simulink model "non_linear_MBS_final"  (R2021b)
settings: Solver=ode15s  StartTime=0  StopTime=30  FixedStep=.00001  RelTol=1e-3
values from neighbouring files (symbols this model uses):
  A_ratio = 1.41    [non_linear_param_MBS_PF_final.m]    (also in non_linear_param_final.m)
  Annulus_area = Bore_area-Rod_area    (= 2356.19449019)    [non_linear_param_MBS_PF_final.m]    (also in non_linear_param_final.m)
  beta_F = 12000e5    (= 1200000000)    [non_linear_param_MBS_PF_final.m]    (also in non_linear_param_final.m)
  Bore_area = pi/4*Bore_diameter^2    (= 3318.30724035)    [non_linear_param_MBS_PF_final.m]    (also in non_linear_param_final.m)
  cyl_c_end = 9e8    (= 900000000)    [non_linear_param_MBS_PF_final.m]    (also in non_linear_param_final.m)
  cyl_c_visc = 1500    [non_linear_param_MBS_PF_final.m]    (also in non_linear_param_final.m)
  cyl_CH_stat = 1/50    (= 0.02)    [non_linear_param_MBS_PF_final.m]    (also in non_linear_param_final.m)
  cyl_F_coul = 75    [non_linear_param_MBS_PF_final.m]    (also in non_linear_param_final.m)
  cyl_FH_stat = 500    [non_linear_param_MBS_PF_final.m]    (also in non_linear_param_final.m)
  cyl_gamma = 250    [non_linear_param_MBS_PF_final.m]    (also in non_linear_param_final.m)
  cyl_k_end = 6e9    (= 6000000000)    [non_linear_param_MBS_PF_final.m]    (also in non_linear_param_final.m)
  cyl_KLi = 0    [non_linear_param_MBS_PF_final.m]    (also in non_linear_param_final.m)
  d_eff_long = sqrt(2*J_sec_long*k_eff_long)    [non_linear_param_MBS_PF_final.m]    (also in non_linear_param_final.m)
  d_eff_short = sqrt(2*J_sec_short*k_eff_short)    [non_linear_param_MBS_PF_final.m]    (also in non_linear_param_final.m)
  DB = 0    [non_linear_param_MBS_PF_final.m]    (also in non_linear_param_final.m = -0.114)
  DR = 1    [non_linear_param_MBS_PF_final.m]    (also in non_linear_param_final.m)
  dr_dcv = 0.7    [non_linear_param_MBS_PF_final.m]    (also in non_linear_param_final.m)
  Eff_Bulk_On = 1    [non_linear_param_MBS_PF_final.m]    (also in non_linear_param_final.m)
  eta_air = 0.7e-2    (= 0.007)    [non_linear_param_MBS_PF_final.m]    (also in non_linear_param_final.m)
  k_cv = 15    [non_linear_param_MBS_PF_final.m]    (also in non_linear_param_final.m)
  k_DCV_PA = 7.85    [non_linear_param_MBS_PF_final.m]    (also in non_linear_param_final.m)
  k_DCV_PB = 7.55    [non_linear_param_MBS_PF_final.m]    (also in non_linear_param_final.m)
  k_DCV_T = 7.85    [non_linear_param_MBS_PF_final.m]    (also in non_linear_param_final.m)
  k_eff_long = E*I/I_sec_long    (= 3050381.94041)    [non_linear_param_MBS_PF_final.m]    (also in non_linear_param_final.m)
  k_eff_short = E*I/I_sec_short    (= 13451432.5427)    [non_linear_param_MBS_PF_final.m]    (also in non_linear_param_final.m)
  k_ocv = 15    [non_linear_param_MBS_PF_final.m]    (also in non_linear_param_final.m)
  K_oil = 1.5e4*0+12000    (= 12000)    [non_linear_param_MBS_PF_final.m]    (also in non_linear_param_final.m)
  k_tanh = 0.005    [non_linear_param_MBS_PF_final.m]    (also in non_linear_param_final.m)
  kappa_air = 1.4    [non_linear_param_MBS_PF_final.m]    (also in non_linear_param_final.m)
  KR = 1    [non_linear_param_MBS_PF_final.m]    (also in non_linear_param_final.m)
  mu_ocv = 10    [non_linear_param_MBS_PF_final.m]    (also in non_linear_param_final.m = 3)
  OL_pa = -0.114    [non_linear_param_MBS_PF_final.m]    (also in non_linear_param_final.m)
  OL_pb = 0.114    [non_linear_param_MBS_PF_final.m]    (also in non_linear_param_final.m)
  p0_A = 62.79    [non_linear_param_MBS_PF_final.m]    (also in non_linear_param_final.m)
  p0_A1 = 1.08    [non_linear_param_MBS_PF_final.m]    (also in non_linear_param_final.m)
  p0_B = 1.213    [non_linear_param_MBS_PF_final.m]    (also in non_linear_param_final.m)
  p_cv_fo = 1    [non_linear_param_MBS_PF_final.m]    (also in non_linear_param_final.m)
  p_cv_set = 2.8    [non_linear_param_MBS_PF_final.m]    (also in non_linear_param_final.m)
  p_ocv_fo = 350    [non_linear_param_MBS_PF_final.m]    (also in non_linear_param_final.m)
  p_ocv_set = 196    [non_linear_param_MBS_PF_final.m]    (also in non_linear_param_final.m = 150)
  p_pc_set = 7    [non_linear_param_MBS_PF_final.m]    (also in non_linear_param_final.m)
  p_R = 1.1    [non_linear_param_MBS_PF_final.m]    (also in non_linear_param_final.m)
  p_S = 180    [non_linear_param_MBS_PF_final.m]    (also in non_linear_param_final.m)
  patm = 1.013e5    (= 101300)    [non_linear_param_MBS_PF_final.m]    (also in non_linear_param_final.m)
  Stroke = 500    [non_linear_param_MBS_PF_final.m]    (also in non_linear_param_final.m)
  t0 = 3    [non_linear_param_MBS_PF_final.m]    (also in non_linear_param_final.m = 1)
  tau_ocv = 0.0889*0+0.001    (= 0.001)    [non_linear_param_MBS_PF_final.m]    (also in non_linear_param_final.m)
  tau_pc = tau_ocv    (= 0.001)    [non_linear_param_MBS_PF_final.m]    (also in non_linear_param_final.m)
  tStepDown = 4.7*0+7.5    (= 7.5)    [non_linear_param_MBS_PF_final.m]    (also in non_linear_param_final.m = 4.6)
  tStepUp = 10.0    (= 10)    [non_linear_param_MBS_PF_final.m]    (also in non_linear_param_final.m = 6.2)
  tWait = 5.0    (= 5)    [non_linear_param_MBS_PF_final.m]    (also in non_linear_param_final.m = 8)
  UL_at = -0.05    [non_linear_param_MBS_PF_final.m]    (also in non_linear_param_final.m)
  UL_bt = 0.05    [non_linear_param_MBS_PF_final.m]    (also in non_linear_param_final.m)
  Up = 1    [non_linear_param_MBS_PF_final.m]    (also in non_linear_param_final.m)
  uS_sp = -0.4    [non_linear_param_MBS_PF_final.m]    (also in non_linear_param_final.m = -0.55)
  V_CH_A = 1.0e-03    (= 0.001)    [non_linear_param_MBS_PF_final.m]    (also in non_linear_param_final.m)
  V_CH_A1 = 0.1e-03    (= 0.0001)    [non_linear_param_MBS_PF_final.m]    (also in non_linear_param_final.m)
  V_CH_B = 1.25e-03    (= 0.00125)    [non_linear_param_MBS_PF_final.m]    (also in non_linear_param_final.m)
  wn_dcv = 30    [non_linear_param_MBS_PF_final.m]    (also in non_linear_param_final.m)
undefined symbols (not defined in the file or in its folder): sigbuilder_block, smiData, uS0, X, Y, Z
libraries: sm_lib x189, nesl_utility x4, eeGeneralControl x2
(part 1 of 6)
settings Model: Creator=Zygis  LastModifiedBy=danielh
settings SolverCC: ZeroCrossAlgorithm=Adaptive  AlgebraicLoopSolver=TrustRegion
settings DataIOCC: ExternalInput=getinputstruct(report1)  InitialState=getstatestruct(report1)  ReturnWorkspaceOutputs=off  Refine=2
settings DebuggingCC: IgnoredZcDiagnostic=none  UniqueDataStoreMsg=error  SFMachineParentedDataDiag=warning  UnderSpecifiedDimensionMsg=warning  ArithmeticOperatorsInVariantConditions=warning
settings HardwareCC: TargetBitPerPointer=64  TargetBitPerSizeT=64  TargetBitPerPtrDiffT=64  TargetLongLongMode=on  TargetIntDivRoundTo=Zero  TargetEndianess=LittleEndian  TargetWordSize=64  TargetHWDeviceType=MATLAB Host  ProdEqTarget=off
settings SFSimCC: SimUseLocalCustomCode=off  CustomCodeUndefinedFunction=UseInterfaceOnly
settings RTWCC: Description=Rapid Simulation Target  SystemTargetFile=rsim.tlc  TemplateMakefile=rsim_default_tmf  GenerateReport=on  RTWUseLocalCustomCode=off
settings RSimTargetCC: TargetLangStandard=C99 (ISO)
settings DiagnosticsConfigSet: SimMechanicsJointTargetOverSpecification=warning

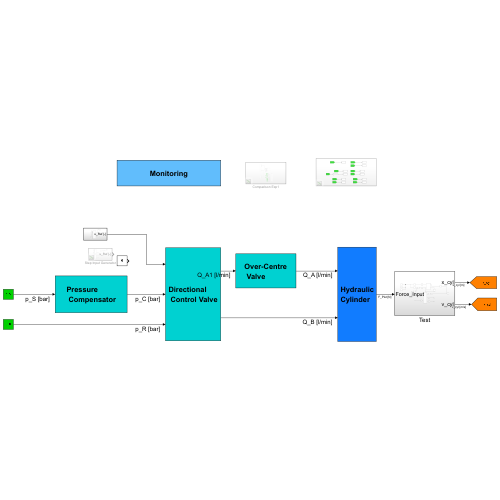
[thumbnail: Simulink's stored preview of the top-level diagram]
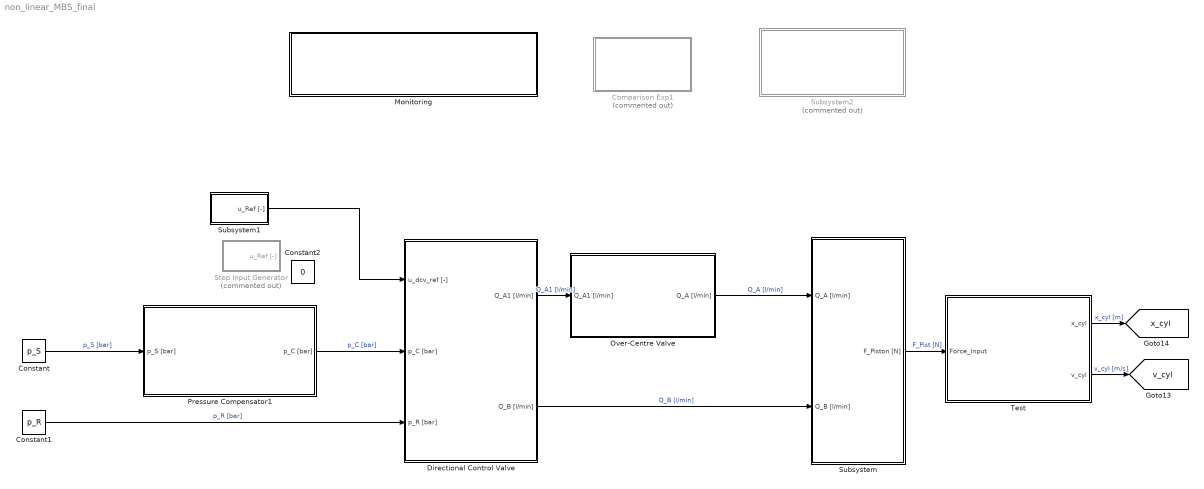
== system non_linear_MBS_final ==
  Comparison Exp1 : Subsystem  Commented=on
  Constant : Constant  Value=p_S
  Constant1 : Constant  Value=p_R
  Constant2 : Constant  Value=0
  Directional Control Valve : Subsystem
      mask Display: disp('{\bf\fontsize{22}Directional\newline Control Valve}', 'texmode','on');
  Monitoring : Subsystem
      mask Display: disp('{\bf\fontsize{22}Monitoring}', 'texmode','on');
  Over-Centre Valve : Subsystem
      mask Display: disp('{\bf\fontsize{22}Over-Centre\newline Valve}', 'texmode','on');
  Pressure Compensator1 : Subsystem
      mask Display: disp('{\bf\fontsize{22}Pressure\newline Compensator}', 'texmode','on');
  Step Input Generator : Subsystem  Commented=on
  Subsystem : Subsystem
      mask Display: disp('{\bf\fontsize{22} Hydraulic \newline Cylinder}', 'texmode','on');
  Subsystem1 : Subsystem
  Subsystem2 : Subsystem  Commented=on
  Test : Subsystem  VariantControl=Flexible_model
  tag scope: v_cyl=global, x_cyl=global
  wires:
  Over-Centre Valve/1(Q_A [l/min]) -> Subsystem/1(Q_A [l/min])  "Q_A [l/min]"
  Directional Control Valve/1(Q_A1 [l/min]) -> Over-Centre Valve/1(Q_A1 [l/min])  "Q_A1 [l/min]"
  Constant/1 -> Pressure Compensator1/1(p_S [bar])  "p_S [bar]"
  Directional Control Valve/2(Q_B [l/min]) -> Subsystem/2(Q_B [l/min])  "Q_B [l/min]"
  Constant1/1 -> Directional Control Valve/3(p_R [bar])  "p_R [bar]"
  Pressure Compensator1/1(p_C [bar]) -> Directional Control Valve/2(p_C [bar])  "p_C [bar]"
  Test/1(x_cyl) -> [x_cyl]  "x_cyl [m]"
  Test/2(v_cyl) -> [v_cyl]  "v_cyl [m/s]"
  Subsystem/1(F_Piston [N]) -> Test/1(Force_Input)  "F_Pist [N]"
  Subsystem1/1(u_Ref [-]) -> Directional Control Valve/1(u_dcv_ref [-])

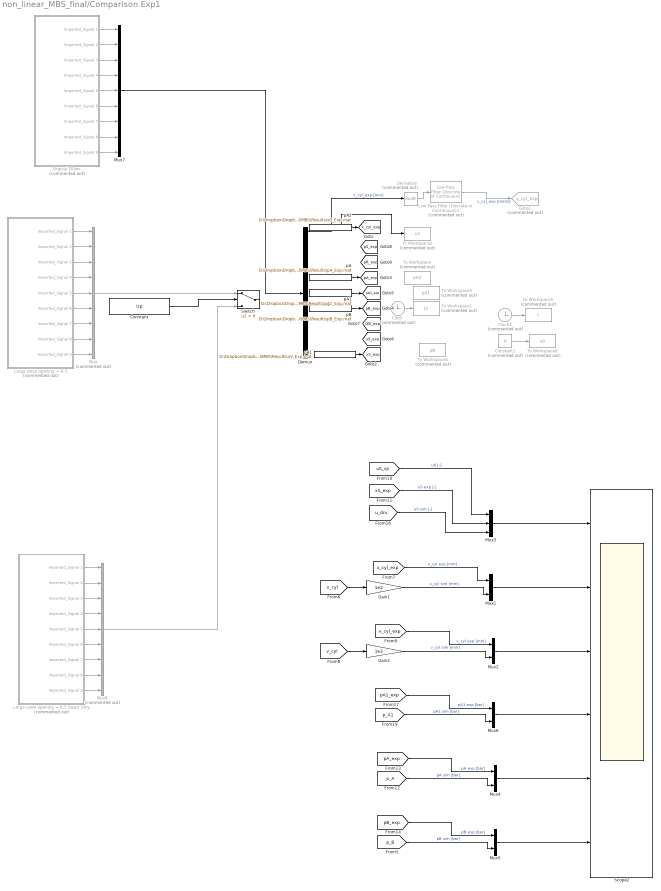
== system non_linear_MBS_final/Comparison Exp1 ==
  Clock : Clock  Commented=on
  Clock1 : Clock  Commented=on
  Constant : Constant  Value=Up
  Constant1 : Constant  Commented=on  Value=0
  Demux : Demux  Outputs=9
  Derivative : Derivative  Commented=on
  Gain1 : Gain  Gain=1e3
  Gain2 : Gain  Gain=1e3
  Goto1 : Goto  Commented=on  GotoTag=v_cyl_exp  TagVisibility=global
  Large valve opening = 0.5 : Subsystem  Tag=STV Subsys  Commented=on  LoadFcn=sigbuilder_block('load');  StartFcn=sigbuilder_block('start');  StopFcn=sigbuilder_block('stop');  ClipboardFcn=sigbuilder_block('clipboard');  mask(Sigbuilder block){tuvar=[0:10]}
      mask help: Simulink:masks:SigbuilderBlock_MD
      mask init:
      | if ~strcmp(get_param(bdroot(gcbh),'SimulationStatus'),'stopped') tuvar = sigbuilder_block('maskInit'); end
      settings: CopyFcn=sigbuilder_block('copy');  DeleteFcn=sigbuilder_block('delete');  ModelCloseFcn=sigbuilder_block('modelClose');  PreSaveFcn=sigbuilder_block('preSave');  PauseFcn=sigbuilder_block('pause');  ContinueFcn=sigbuilder_block('continue');  NameChangeFcn=sigbuilder_block('namechange');  OpenFcn=sigbuilder_block('open',[41.25 0 1392.75 801.75 ]);  CloseFcn=sigbuilder_block('close');
      mask Display: plot(0, 0, 100, 100,[2, 2, 32, 32, 2], [68, 8, 8, 68, 68],[32, 2], [38, 38], [32, 19, 2],[53, 60, 44], [32, 17, 17, 2],[16, 16, 31, 31]);txt = getActiveGroup(gcbh);text(2, 100, txt,'verticalAlignment', 'top');
  Large valve opening = 0.5 Down Only : Subsystem  Tag=STV Subsys  Commented=on  LoadFcn=sigbuilder_block('load');  StartFcn=sigbuilder_block('start');  StopFcn=sigbuilder_block('stop');  ClipboardFcn=sigbuilder_block('clipboard');  mask(Sigbuilder block){tuvar=[0:10]}
      mask help: Simulink:masks:SigbuilderBlock_MD
      mask init:
      | if ~strcmp(get_param(bdroot(gcbh),'SimulationStatus'),'stopped') tuvar = sigbuilder_block('maskInit'); end
      settings: CopyFcn=sigbuilder_block('copy');  DeleteFcn=sigbuilder_block('delete');  ModelCloseFcn=sigbuilder_block('modelClose');  PreSaveFcn=sigbuilder_block('preSave');  PauseFcn=sigbuilder_block('pause');  ContinueFcn=sigbuilder_block('continue');  NameChangeFcn=sigbuilder_block('namechange');  OpenFcn=sigbuilder_block('open',[41.25 0 1392.75 801.75 ]);  CloseFcn=sigbuilder_block('close');
      mask Display: plot(0, 0, 100, 100,[2, 2, 32, 32, 2], [68, 8, 8, 68, 68],[32, 2], [38, 38], [32, 19, 2],[53, 60, 44], [32, 17, 17, 2],[16, 16, 31, 31]);txt = getActiveGroup(gcbh);text(2, 100, txt,'verticalAlignment', 'top');
  Low-Pass Filter (Discrete or Continuous)2 : eeGeneralControl/Low-Pass Filter (Discrete or Continuous)  Commented=on  K=1  T=0.05  B=150  A=-150  init_option=Inherited from block input  x0=0  Ts=0.001
  Mux : Mux  Commented=on  Inputs=9
  Mux{1..2,4..6} (5 alike) : Mux  Inputs=2
  Mux3 : Mux  Inputs=3
  Mux7 : Mux  Inputs=9
  Mux8 : Mux  Commented=on  Inputs=9
  Orginal DOwn : Subsystem  Tag=STV Subsys  Commented=on  LoadFcn=sigbuilder_block('load');  StartFcn=sigbuilder_block('start');  StopFcn=sigbuilder_block('stop');  ClipboardFcn=sigbuilder_block('clipboard');  mask(Sigbuilder block){tuvar=[0:10]}
      mask help: Simulink:masks:SigbuilderBlock_MD
      mask init:
      | if ~strcmp(get_param(bdroot(gcbh),'SimulationStatus'),'stopped') tuvar = sigbuilder_block('maskInit'); end
      settings: CopyFcn=sigbuilder_block('copy');  DeleteFcn=sigbuilder_block('delete');  ModelCloseFcn=sigbuilder_block('modelClose');  PreSaveFcn=sigbuilder_block('preSave');  PauseFcn=sigbuilder_block('pause');  ContinueFcn=sigbuilder_block('continue');  NameChangeFcn=sigbuilder_block('namechange');  OpenFcn=sigbuilder_block('open',[437.6 199.2 550.4 400 ]);  CloseFcn=sigbuilder_block('close');
      mask Display: plot(0, 0, 100, 100,[2, 2, 32, 32, 2], [68, 8, 8, 68, 68],[32, 2], [38, 38], [32, 19, 2],[53, 60, 44], [32, 17, 17, 2],[16, 16, 31, 31]);txt = getActiveGroup(gcbh);text(2, 100, txt,'verticalAlignment', 'top');
  Scope2 : Scope  NumInputPorts=6
  Switch : Switch  Criteria=u2 > Threshold
  To Workspace : ToWorkspace  Commented=on  VariableName=pA2  SampleTime=0.001
      settings: Save2DSignal=2-D array (concatenate along first dimension)
  To Workspace1 : ToWorkspace  Commented=on  VariableName=t2  SampleTime=0.001
      settings: Save2DSignal=2-D array (concatenate along first dimension)
  To Workspace2 : ToWorkspace  Commented=on  VariableName=x2  SampleTime=0.001
      settings: Save2DSignal=2-D array (concatenate along first dimension)
  To Workspace3 : ToWorkspace  Commented=on  VariableName=pA1  SampleTime=0.001
      settings: Save2DSignal=2-D array (concatenate along first dimension)
  To Workspace4 : ToWorkspace  Commented=on  VariableName=pB  SampleTime=0.001
      settings: Save2DSignal=2-D array (concatenate along first dimension)
  To Workspace5 : ToWorkspace  Commented=on  VariableName=t  SampleTime=0.001
      settings: Save2DSignal=2-D array (concatenate along first dimension)
  To Workspace6 : ToWorkspace  Commented=on  VariableName=v0  SampleTime=0.001
      settings: Save2DSignal=2-D array (concatenate along first dimension)
  pA : FromFile  FileName=D:\Dropbox\Dropbox\PhD\Models\LSS model\Comparison_Paper_Model\Non Linear Model\MBS\Results\pA_Exp.mat  SampleTime=0
  pA1 : FromFile  FileName=D:\Dropbox\Dropbox\PhD\Models\LSS model\Comparison_Paper_Model\Non Linear Model\MBS\Results\pA1_Exp.mat  SampleTime=0
  pA2 : FromFile  FileName=D:\Dropbox\Dropbox\PhD\Models\LSS model\Comparison_Paper_Model\Non Linear Model\MBS\Results\xC_Exp.mat  SampleTime=0
  pB : FromFile  FileName=D:\Dropbox\Dropbox\PhD\Models\LSS model\Comparison_Paper_Model\Non Linear Model\MBS\Results\pB_Exp.mat  SampleTime=0
  pB1 : FromFile  FileName=D:\Dropbox\Dropbox\PhD\Models\LSS model\Comparison_Paper_Model\Non Linear Model\MBS\Results\xV_Exp.mat  SampleTime=0
  tag scope: QB_exp=global, pA1_exp=global, pA_exp=global, pB_exp=global, pR_exp=global, pS_exp=global, uS_exp=global, xS_exp=global, x_cyl_exp=global
  wires:
  Demux/1 -> To Workspace2/1, Derivative/1  "x_cyl_exp [mm]"
  Low-Pass Filter (Discrete or Continuous)2/1 -> Goto1/1  "v_cyl_exp [mm/s]"
  Derivative/1 -> Low-Pass Filter (Discrete or Continuous)2/1
  Large valve opening = 0.5/4(Imported_Signal 4) -> Mux/4
  Large valve opening = 0.5/5(Imported_Signal 5) -> Mux/5
  Large valve opening = 0.5/6(Imported_Signal 6) -> Mux/6
  Large valve opening = 0.5/7(Imported_Signal 7) -> Mux/7
  Large valve opening = 0.5/8(Imported_Signal 8) -> Mux/8
  Large valve opening = 0.5/9(Imported_Signal 9) -> Mux/9
  Large valve opening = 0.5/3(Imported_Signal 3) -> Mux/3
  Large valve opening = 0.5/1(Imported_Signal 1) -> Mux/1
  Large valve opening = 0.5/2(Imported_Signal 2) -> Mux/2
  Mux/1 -> Switch/1
  Constant/1 -> Switch/2
  [x_cyl] -> Gain1/1
  [p_A] -> Mux4/2  "pA sim [bar]"
  [v_cyl] -> Gain2/1
  Mux4/1 -> Scope2/5
  Mux2/1 -> Scope2/3
  [xS_exp] -> Mux3/2  "xS exp [-]"
  [pA_exp] -> Mux4/1  "pA exp [bar]"
  Gain1/1 -> Mux1/2  "x_cyl sim [mm]"
  Gain2/1 -> Mux2/2  "v_cyl sim [mm]"
  [p_A1] -> Mux6/2  "pA1 sim [bar]"
  [pB_exp] -> Mux5/1  "pB exp [bar]"
  [u_dcv] -> Mux3/3  "xS sim [-]"
  Mux3/1 -> Scope2/1
  [uS_sp] -> Mux3/1  "uS [-]"
  [p_B] -> Mux5/2  "pB sim [bar]"
  Mux6/1 -> Scope2/4
  Mux1/1 -> Scope2/2
  [v_cyl_exp] -> Mux2/1  "v_cyl exp [mm]"
  [pA1_exp] -> Mux6/1  "pA1 exp [bar]"
  Mux5/1 -> Scope2/6
  Large valve opening = 0.5 Down Only/4(Imported_Signal 4) -> Mux8/4
  Large valve opening = 0.5 Down Only/8(Imported_Signal 8) -> Mux8/8
  Large valve opening = 0.5 Down Only/1(Imported_Signal 1) -> Mux8/1
  Large valve opening = 0.5 Down Only/2(Imported_Signal 2) -> Mux8/2
  Large valve opening = 0.5 Down Only/6(Imported_Signal 6) -> Mux8/6
  Large valve opening = 0.5 Down Only/9(Imported_Signal 9) -> Mux8/9
  Large valve opening = 0.5 Down Only/3(Imported_Signal 3) -> Mux8/3
  Large valve opening = 0.5 Down Only/5(Imported_Signal 5) -> Mux8/5
  Large valve opening = 0.5 Down Only/7(Imported_Signal 7) -> Mux8/7
  Mux8/1 -> Switch/3
  Orginal DOwn/1(Imported_Signal 1) -> Mux7/1
  Orginal DOwn/8(Imported_Signal 8) -> Mux7/8
  Orginal DOwn/2(Imported_Signal 2) -> Mux7/2
  Orginal DOwn/9(Imported_Signal 9) -> Mux7/9
  Orginal DOwn/6(Imported_Signal 6) -> Mux7/6
  Orginal DOwn/5(Imported_Signal 5) -> Mux7/5
  Orginal DOwn/3(Imported_Signal 3) -> Mux7/3
  Orginal DOwn/7(Imported_Signal 7) -> Mux7/7
  Orginal DOwn/4(Imported_Signal 4) -> Mux7/4
  Clock/1 -> To Workspace1/1
  Mux7/1 -> Demux/1
  Clock1/1 -> To Workspace5/1
  Constant1/1 -> To Workspace6/1
  [x_cyl_exp] -> Mux1/1  "x_cyl exp [mm]"
  pA/1 -> [pA_exp]
  pA1/1 -> [pA1_exp]
  pB/1 -> [pB_exp]
  pB1/1 -> [xS_exp]
  pA2/1 -> [x_cyl_exp]

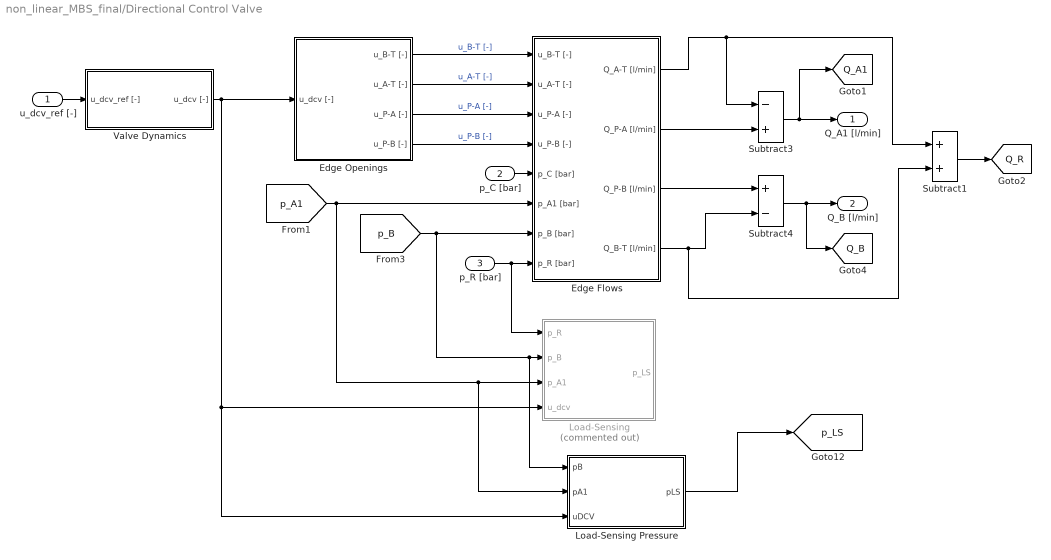
== system non_linear_MBS_final/Directional Control Valve ==
  u_dcv_ref [-] : Inport
  p_C [bar] : Inport  Port=2
  p_R [bar] : Inport  Port=3
  Edge Flows : Subsystem
  Edge Openings : Subsystem
  Load-Sensing : MATLAB Function  Commented=on  TreatAsAtomicUnit=on
  Load-Sensing Pressure : Subsystem
  Subtract1 : Sum
  Subtract3 : Sum  Inputs=-+
  Subtract4 : Sum  Inputs=+-
  Valve Dynamics : Subsystem
  Q_A1 [l/min] : Outport  VectorParamsAs1DForOutWhenUnconnected=off
  Q_B [l/min] : Outport  Port=2  VectorParamsAs1DForOutWhenUnconnected=off
  tag scope: Q_A1=global, Q_B=global, Q_R=global, p_LS=global
  wires:
  Edge Flows/2(Q_P-A [l/min]) -> Subtract3/2
  u_dcv_ref [-]/1 -> Valve Dynamics/1(u_dcv_ref [-])
  p_R [bar]/1 -> Load-Sensing/1(p_R), Edge Flows/8(p_R [bar])
  [p_A1] -> Load-Sensing Pressure/2(pA1), Load-Sensing/3(p_A1), Edge Flows/6(p_A1 [bar])
  Subtract3/1 -> [Q_A1], Q_A1 [l/min]/1
  Subtract4/1 -> [Q_B], Q_B [l/min]/1
  [p_B] -> Load-Sensing Pressure/1(pB), Load-Sensing/2(p_B), Edge Flows/7(p_B [bar])
  p_C [bar]/1 -> Edge Flows/5(p_C [bar])
  Edge Flows/4(Q_B-T [l/min]) -> Subtract1/2, Subtract4/2
  Subtract1/1 -> [Q_R]
  Edge Openings/2(u_A-T [-]) -> Edge Flows/2(u_A-T [-])  "u_A-T [-]"
  Edge Openings/4(u_P-B [-]) -> Edge Flows/4(u_P-B [-])  "u_P-B [-]"
  Edge Openings/1(u_B-T [-]) -> Edge Flows/1(u_B-T [-])  "u_B-T [-]"
  Edge Openings/3(u_P-A [-]) -> Edge Flows/3(u_P-A [-])  "u_P-A [-]"
  Edge Flows/3(Q_P-B [l/min]) -> Subtract4/1
  Edge Flows/1(Q_A-T [l/min]) -> Subtract3/1, Subtract1/1
  Valve Dynamics/1(u_dcv [-]) -> Load-Sensing Pressure/3(uDCV), Load-Sensing/4(u_dcv), Edge Openings/1(u_dcv [-])
  Load-Sensing Pressure/1(pLS) -> [p_LS]

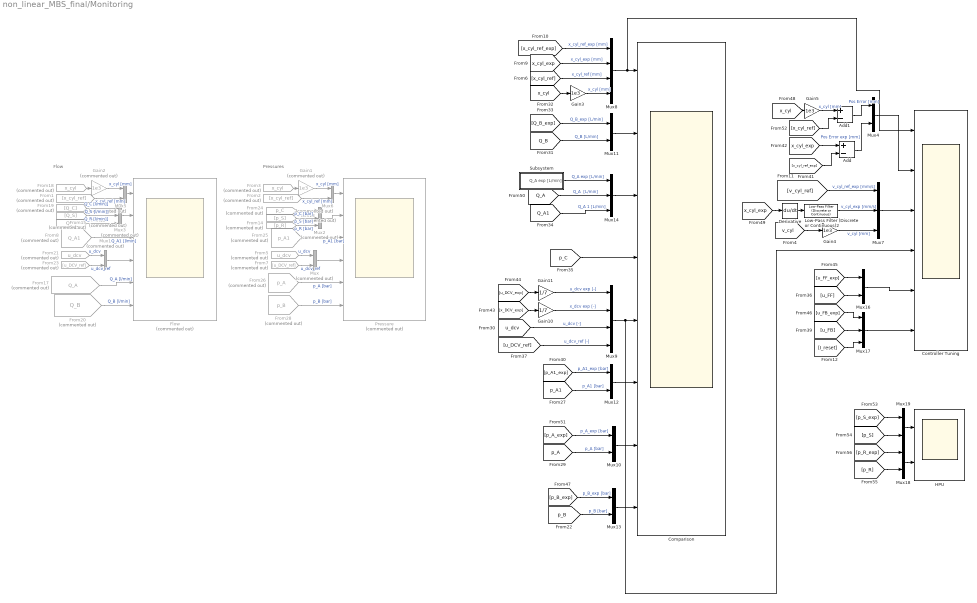
== system non_linear_MBS_final/Monitoring ==
  note: Pressures
  note: Flow
  Add : Sum  Inputs=+-
  Add1 : Sum  Inputs=+-
  Comparison : Scope  NumInputPorts=8
  Controller Tuning : Scope  NumInputPorts=6
  Derivative : Derivative
  Flow : Scope  Commented=on  NumInputPorts=6
  From1 : From  Commented=on  GotoTag=x_cyl_ref  TagVisibility=global
  From13 : From  Commented=on  GotoTag=p_S  TagVisibility=global
  From14 : From  Commented=on  GotoTag=p_R  TagVisibility=global
  From15 : From  Commented=on  GotoTag=Q_S  TagVisibility=global
  From16 : From  Commented=on  GotoTag=Q_R  TagVisibility=global
  From17 : From  Commented=on  GotoTag=Q_A  TagVisibility=global
  From18 : From  Commented=on  GotoTag=x_cyl  TagVisibility=global
  From19 : From  Commented=on  GotoTag=Q_C  TagVisibility=global
  From2 : From  Commented=on  GotoTag=x_cyl_ref  TagVisibility=global
  From20 : From  Commented=on  GotoTag=Q_B  TagVisibility=global
  From21 : From  Commented=on  GotoTag=u_dcv  TagVisibility=global
  From23 : From  Commented=on  GotoTag=u_DCV_ref  TagVisibility=global
  From24 : From  Commented=on  GotoTag=p_C  TagVisibility=global
  From25 : From  Commented=on  GotoTag=p_A1  TagVisibility=global
  From26 : From  Commented=on  GotoTag=p_A  TagVisibility=global
  From28 : From  Commented=on  GotoTag=p_B  TagVisibility=global
  From3 : From  Commented=on  GotoTag=x_cyl  TagVisibility=global
  From5 : From  Commented=on  GotoTag=u_dcv  TagVisibility=global
  From7 : From  Commented=on  GotoTag=u_DCV_ref  TagVisibility=global
  From8 : From  Commented=on  GotoTag=Q_A1  TagVisibility=global
  Gain1 : Gain  Commented=on  Gain=1e3
  Gain10 : Gain  Gain=1/7
  Gain11 : Gain  Gain=1/7
  Gain2 : Gain  Commented=on  Gain=1e3
  Gain3 : Gain  Gain=1e3
  Gain4 : Gain  Gain=1e3
  Gain5 : Gain  Gain=1e3
  HPU : Scope  NumInputPorts=2
  Low-Pass Filter (Discrete or Continuous)2 : eeGeneralControl/Low-Pass Filter (Discrete or Continuous)  K=1  T=0.1  B=150  A=-150  init_option=Inherited from block input  x0=0  Ts=0
  Mux{,1,5..6} (4 alike) : Mux  Commented=on  Inputs=2
  Mux{4,10..13,16,18..19} (8 alike) : Mux  Inputs=2
  Mux14 : Mux  Inputs=3
  Mux17 : Mux  Inputs=3
  Mux2 : Mux  Commented=on  Inputs=3
  Mux3 : Mux  Commented=on  Inputs=3
  Mux7 : Mux  Inputs=3
  Mux8 : Mux
  Mux9 : Mux
  Pressure : Scope  Commented=on  NumInputPorts=6
  Subsystem : Subsystem
  wires:
  From28/1 -> Pressure/6  "p_B [bar]"
  From8/1 -> Flow/3  "Q_A1 [l/min]"
  From21/1 -> Mux1/1  "u_dcv"
  From17/1 -> Flow/5  "Q_A [l/min]"
  From26/1 -> Pressure/5  "p_A [bar]"
  From23/1 -> Mux1/2  "u_dcv_ref"
  From20/1 -> Flow/6  "Q_B [l/min]"
  Mux/1 -> Pressure/4
  From2/1 -> Mux6/2  "x_cyl_ref [mm]"
  From16/1 -> Mux3/3  "Q_R [l/min]]"
  From3/1 -> Gain1/1
  From24/1 -> Mux2/1  "p_C [bar]"
  From7/1 -> Mux/2  "u_dcv_ref"
  Mux1/1 -> Flow/4
  From25/1 -> Pressure/3  "p_A1 [bar]"
  Mux3/1 -> Flow/2
  Mux2/1 -> Pressure/2
  From5/1 -> Mux/1  "u_dcv"
  Mux5/1 -> Flow/1
  From14/1 -> Mux2/3  "p_R [bar]"
  From1/1 -> Mux5/2  "x_cyl_ref [mm]"
  From15/1 -> Mux3/2  "Q_S [l/min]]"
  From18/1 -> Gain2/1
  Gain2/1 -> Mux5/1  "x_cyl [mm]"
  From19/1 -> Mux3/1  "Q_C [l/min]]"
  From13/1 -> Mux2/2  "p_S [bar]"
  Gain1/1 -> Mux6/1  "x_cyl [mm]"
  Mux6/1 -> Pressure/1
  Mux8/1 -> Controller Tuning/1, Comparison/1
  [x_cyl_ref] -> Mux8/3  "x_cyl_ref [mm]"
  [x_cyl] -> Gain3/1
  Gain3/1 -> Mux8/4  "x_cyl [mm]"
  [x_cyl_ref_exp] -> Mux8/1  "x_cyl_ref_exp [mm]"
  [x_cyl_exp] -> Mux8/2  "x_cyl_exp [mm]"
  [Q_B] -> Mux11/2  "Q_B [L/min]"
  [Q_B_exp] -> Mux11/1  "Q_B_exp [L/min]"
  Mux11/1 -> Comparison/2
  [p_R] -> Mux18/2
  [p_R_exp] -> Mux18/1
  Mux18/1 -> HPU/2
  [p_S_exp] -> Mux19/1
  [p_S] -> Mux19/2
  Mux19/1 -> HPU/1
  [p_C] -> Comparison/4
  Mux17/1 -> Controller Tuning/6
  [u_FB] -> Mux17/2
  [u_FF] -> Mux16/2
  [u_FF_exp] -> Mux16/1
  [u_FB_exp] -> Mux17/1
  Mux16/1 -> Controller Tuning/5
  [x_cyl_exp] -> Add/1
  [x_cyl_ref] -> Add1/2
  [x_cyl_ref_exp] -> Add/2
  Gain5/1 -> Add1/1  "x_cyl [mm]"
  [x_cyl] -> Gain5/1
  Add/1 -> Mux4/2  "Pos Error exp [mm]"
  Add1/1 -> Mux4/1  "Pos Error [mm]"
  Mux4/1 -> Controller Tuning/2
  [I_reset] -> Mux17/3
  [x_DCV_exp] -> Gain10/1
  Gain10/1 -> Mux9/2  "x_dcv exp [-]"
  [u_DCV_ref] -> Mux9/4  "u_dcv_ref [-]"
  [u_dcv] -> Mux9/3  "u_dcv [-]"
  Gain11/1 -> Mux9/1  "u_dcv exp [-]"
  [p_A1_exp] -> Mux12/1  "p_A1_exp [bar]"
  [p_A1] -> Mux12/2  "p_A1 [bar]"
  Mux12/1 -> Comparison/6
  Mux9/1 -> Controller Tuning/4, Comparison/5
  Low-Pass Filter (Discrete or Continuous)2/1 -> Mux7/2  "v_cyl_exp [mm/s]"
  Mux7/1 -> Controller Tuning/3
  Derivative/1 -> Low-Pass Filter (Discrete or Continuous)2/1
  [v_cyl_ref] -> Mux7/1  "v_cyl_ref_exp [mm/s]"
  [v_cyl] -> Gain4/1
  [x_cyl_exp] -> Derivative/1
  Gain4/1 -> Mux7/3  "v_cyl [mm]"
  [Q_A1] -> Mux14/3  "Q_A 1 [L/min]"
  Subsystem/1(Q_A exp [L/min]) -> Mux14/1  "Q_A exp [L/min]"
  [Q_A] -> Mux14/2  "Q_A  [L/min]"
  Mux14/1 -> Comparison/3
  [u_DCV_exp] -> Gain11/1
  [p_B] -> Mux13/2  "p_B [bar]"
  [p_B_exp] -> Mux13/1  "p_B_exp [bar]"
  [p_A] -> Mux10/2  "p_A [bar]"
  [p_A_exp] -> Mux10/1  "p_A_exp [bar]"
  Mux13/1 -> Comparison/8
  Mux10/1 -> Comparison/7

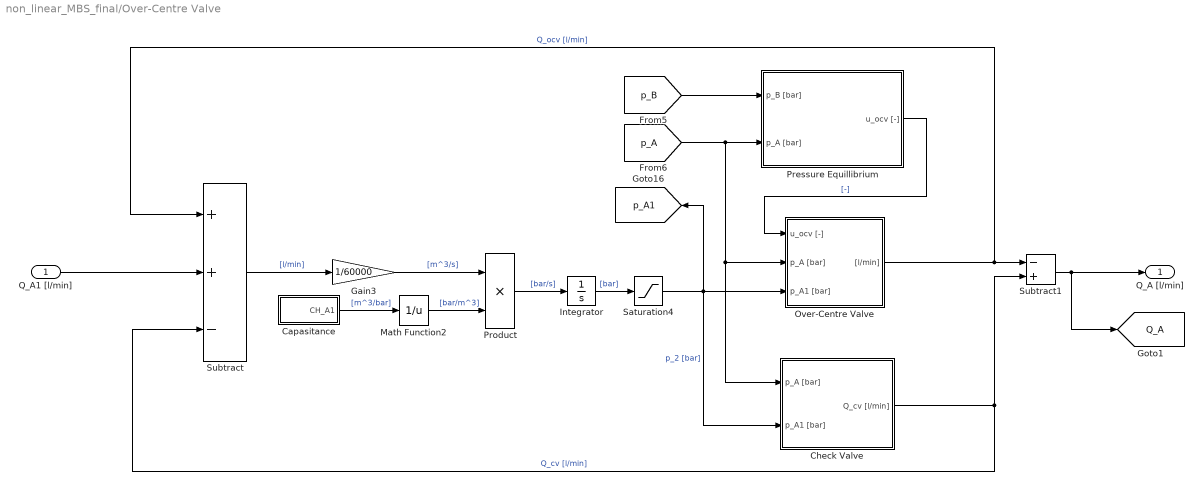
== system non_linear_MBS_final/Over-Centre Valve ==
  Q_A1 [l/min] : Inport
  Capasitance : Subsystem
  Check Valve : Subsystem
  Gain3 : Gain  Gain=1/60000
  Integrator : Integrator  InitialCondition=p0_A1
  Math Function2 : Math  Operator=reciprocal  SignedPower=on  SaturateOnIntegerOverflow=on
  Over-Centre Valve : Subsystem
  Pressure Equillibrium : Subsystem
  Product : Product
  Saturation4 : Saturate  UpperLimit=200  LowerLimit=p_R  ZeroCross=off
  Subtract : Sum  Inputs=++-
  Subtract1 : Sum  Inputs=-+
  Q_A [l/min] : Outport  PortDimensions=1  VectorParamsAs1DForOutWhenUnconnected=off
  tag scope: Q_A=global, p_A1=global
  wires:
  [p_B] -> Pressure Equillibrium/1(p_B [bar])
  Pressure Equillibrium/1(u_ocv [-]) -> Over-Centre Valve/1(u_ocv [-])  "[-]"
  Check Valve/1(Q_cv [l/min]) -> Subtract/3, Subtract1/2  "Q_cv [l/min]"
  Over-Centre Valve/1("[l/min]") -> Subtract1/1, Subtract/1  "Q_ocv [l/min]"
  Saturation4/1 -> Check Valve/2(p_A1 [bar]), Over-Centre Valve/3(p_A1 [bar]), [p_A1]  "p_2 [bar]"
  Subtract/1 -> Gain3/1  "[l/min]"
  Q_A1 [l/min]/1 -> Subtract/2
  Product/1 -> Integrator/1  "[bar/s]"
  Integrator/1 -> Saturation4/1  "[bar]"
  Gain3/1 -> Product/1  "[m^3/s]"
  Subtract1/1 -> [Q_A], Q_A [l/min]/1
  [p_A] -> Pressure Equillibrium/2(p_A [bar]), Over-Centre Valve/2(p_A [bar]), Check Valve/1(p_A [bar])
  Capasitance/1("CH_A1 ") -> Math Function2/1  "[m^3/bar]"
  Math Function2/1 -> Product/2  "[bar/m^3]"

== system non_linear_MBS_final/Pressure Compensator1 ==
  p_S [bar] : Inport
  Compensator From Morten Bak Paper : MATLAB Function  TreatAsAtomicUnit=on
  "Compensator setting [bar] " : Constant  Value=p_pc_set
  Transfer Fcn1 : TransferFcn  Denominator=[tau_pc 1]
  p_C [bar] : Outport  VectorParamsAs1DForOutWhenUnconnected=off
  tag scope: p_C=global
  wires:
  [p_LS] -> Compensator From Morten Bak Paper/3(p_LS)
  "Compensator setting [bar] "/1 -> Compensator From Morten Bak Paper/1(p0)
  p_S [bar]/1 -> Compensator From Morten Bak Paper/2(p_S)
  Compensator From Morten Bak Paper/1(p_C) -> Transfer Fcn1/1  "p_C [bar]"
  Transfer Fcn1/1 -> [p_C], p_C [bar]/1  "u_ocv_ref [-]"

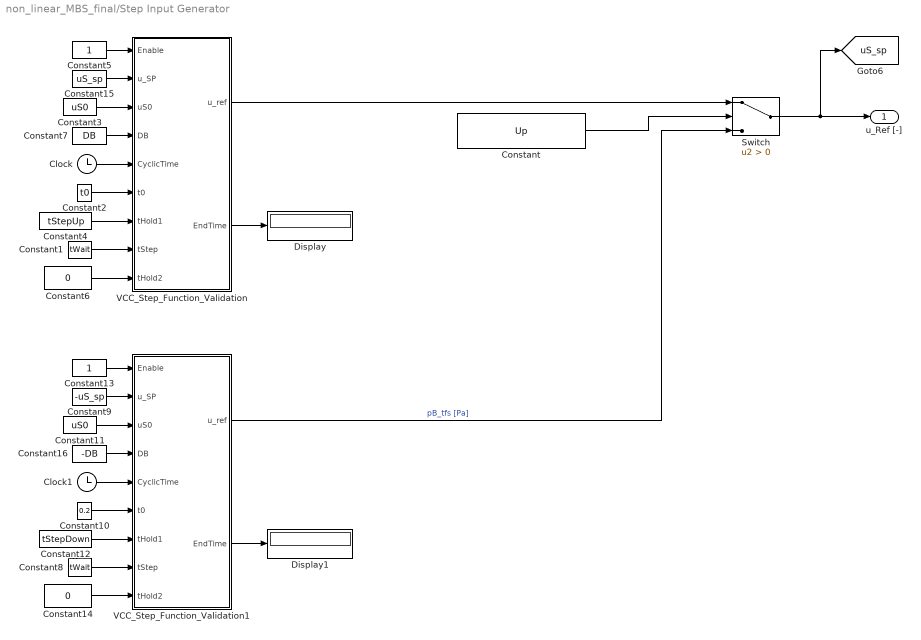
== system non_linear_MBS_final/Step Input Generator ==
  Clock : Clock
  Clock1 : Clock
  Constant : Constant  Value=Up
  Constant1 : Constant  Value=tWait
  Constant10 : Constant  Value=0.2
  Constant11 : Constant  Value=uS0
  Constant12 : Constant  Value=tStepDown
  Constant13 : Constant  OutDataTypeStr=boolean
  Constant14 : Constant  Value=0
  Constant15 : Constant  Value=uS_sp
  Constant16 : Constant  Value=-DB
  Constant2 : Constant  Value=t0
  Constant3 : Constant  Value=uS0
  Constant4 : Constant  Value=tStepUp
  Constant5 : Constant  OutDataTypeStr=boolean
  Constant6 : Constant  Value=0
  Constant7 : Constant  Value=DB
  Constant8 : Constant  Value=tWait
  Constant9 : Constant  Value=-uS_sp
  Display : Display
  Display1 : Display
  Switch : Switch  Criteria=u2 > Threshold
  VCC_Step_Function_Validation : Subsystem  TreatAsAtomicUnit=on
  VCC_Step_Function_Validation1 : Subsystem  TreatAsAtomicUnit=on
  u_Ref [-] : Outport  VectorParamsAs1DForOutWhenUnconnected=off
  tag scope: uS_sp=global
  wires:
  VCC_Step_Function_Validation/2(EndTime) -> Display/1
  Constant4/1 -> VCC_Step_Function_Validation/7(tHold1)
  Constant1/1 -> VCC_Step_Function_Validation/8(tStep)
  Clock/1 -> VCC_Step_Function_Validation/5(CyclicTime)
  Constant2/1 -> VCC_Step_Function_Validation/6(t0)
  Constant15/1 -> VCC_Step_Function_Validation/2(u_SP)
  VCC_Step_Function_Validation/1(u_ref) -> Switch/1
  Constant3/1 -> VCC_Step_Function_Validation/3(uS0)
  Constant6/1 -> VCC_Step_Function_Validation/9(tHold2)
  Constant5/1 -> VCC_Step_Function_Validation/1(Enable)
  Constant7/1 -> VCC_Step_Function_Validation/4(DB)
  Switch/1 -> [uS_sp], u_Ref [-]/1
  Constant/1 -> Switch/2
  VCC_Step_Function_Validation1/1(u_ref) -> Switch/3  "pB_tfs [Pa]"
  Constant16/1 -> VCC_Step_Function_Validation1/4(DB)
  Constant13/1 -> VCC_Step_Function_Validation1/1(Enable)
  Constant11/1 -> VCC_Step_Function_Validation1/3(uS0)
  Constant12/1 -> VCC_Step_Function_Validation1/7(tHold1)
  Clock1/1 -> VCC_Step_Function_Validation1/5(CyclicTime)
  VCC_Step_Function_Validation1/2(EndTime) -> Display1/1
  Constant9/1 -> VCC_Step_Function_Validation1/2(u_SP)
  Constant14/1 -> VCC_Step_Function_Validation1/9(tHold2)
  Constant10/1 -> VCC_Step_Function_Validation1/6(t0)
  Constant8/1 -> VCC_Step_Function_Validation1/8(tStep)

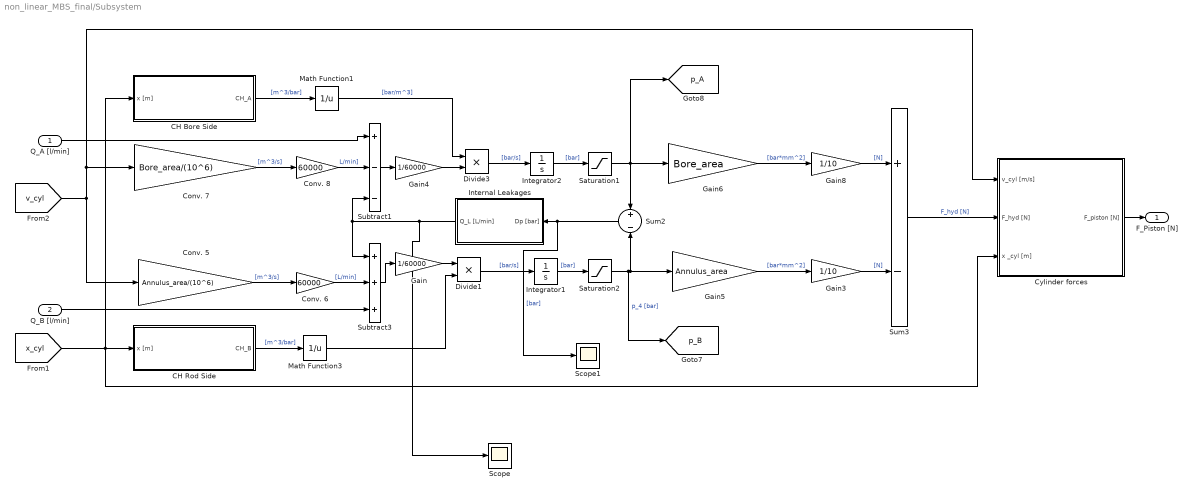
== system non_linear_MBS_final/Subsystem ==
  Q_A [l/min] : Inport
  Q_B [l/min] : Inport  Port=2
  CH Bore Side : Subsystem
  CH Rod Side : Subsystem
  Conv. 5 : Gain  Gain=Annulus_area/(10^6)
  Conv. 6 : Gain  Gain=60000
  Conv. 7 : Gain  Gain=Bore_area/(10^6)
  Conv. 8 : Gain  Gain=60000
  Cylinder forces : Subsystem
  Divide1 : Product  Inputs=**
  Divide3 : Product  Inputs=**
  Gain : Gain  Gain=1/60000
  Gain3 : Gain  Gain=1/10
  Gain4 : Gain  Gain=1/60000
  Gain5 : Gain  Gain=Annulus_area
  Gain6 : Gain  Gain=Bore_area
  Gain8 : Gain  Gain=1/10
  Integrator1 : Integrator  InitialCondition=p0_B  LowerSaturationLimit=0
  Integrator2 : Integrator  InitialCondition=p0_A  LowerSaturationLimit=0
  Internal Leakages : Subsystem
  Math Function1 : Math  Operator=reciprocal  SignedPower=on  SaturateOnIntegerOverflow=on
  Math Function3 : Math  Operator=reciprocal  SignedPower=on  SaturateOnIntegerOverflow=on
  Saturation1 : Saturate  UpperLimit=200  LowerLimit=p_R  ZeroCross=off
  Saturation2 : Saturate  UpperLimit=200  LowerLimit=p_R  ZeroCross=off
  Scope : Scope
  Scope1 : Scope
  Subtract1 : Sum  Inputs=+--
  Subtract3 : Sum  Inputs=+++
  Sum2 : Sum  Inputs=+-
  Sum3 : Sum  Inputs=+-
  F_Piston [N] : Outport  VectorParamsAs1DForOutWhenUnconnected=off
  tag scope: p_A=global, p_B=global
  wires:
  Sum3/1 -> Cylinder forces/2(F_hyd [N])  "F_hyd [N]"
  Gain8/1 -> Sum3/1  "[N]"
  Gain3/1 -> Sum3/2  "[N]"
  Saturation1/1 -> Gain6/1, [p_A], Sum2/1
  Gain5/1 -> Gain3/1  "[bar*mm^2]"
  Gain6/1 -> Gain8/1  "[bar*mm^2]"
  Cylinder forces/1(F_piston [N]) -> F_Piston [N]/1
  [v_cyl] -> Cylinder forces/1(v_cyl [m/s]), Conv. 7/1, Conv. 5/1
  [x_cyl] -> CH Rod Side/1(x [m]), CH Bore Side/1(x [m]), Cylinder forces/3(x _cyl [m])
  Integrator1/1 -> Saturation2/1  "[bar]"
  Q_B [l/min]/1 -> Subtract3/3
  Divide1/1 -> Integrator1/1  "[bar/s]"
  Saturation2/1 -> Sum2/2, Gain5/1, [p_B]  "p_4 [bar]"
  Gain/1 -> Divide1/1
  Integrator2/1 -> Saturation1/1  "[bar]"
  Gain4/1 -> Divide3/2
  Divide3/1 -> Integrator2/1  "[bar/s]"
  Math Function1/1 -> Divide3/1  "[bar/m^3]"
  CH Bore Side/1(CH_A) -> Math Function1/1  "[m^3/bar]"
  Math Function3/1 -> Divide1/2
  CH Rod Side/1(CH_B) -> Math Function3/1  "[m^3/bar]"
  Subtract3/1 -> Gain/1
  Conv. 6/1 -> Subtract3/2  "[L/min]"
  Conv. 5/1 -> Conv. 6/1  "[m^3/s]"
  Subtract1/1 -> Gain4/1
  Q_A [l/min]/1 -> Subtract1/1
  Conv. 7/1 -> Conv. 8/1  "[m^3/s]"
  Conv. 8/1 -> Subtract1/2  "L/min]"
  Sum2/1 -> Scope1/1, Internal Leakages/1(Dp [bar])  "[bar]"
  Internal Leakages/1(Q_L [L/min]) -> Scope/1, Subtract3/1, Subtract1/3

== system non_linear_MBS_final/Subsystem1 ==
  Clock : Clock
  Constant1 : Constant  Value=tWait
  Constant15 : Constant  Value=uS_sp
  Constant2 : Constant  Value=t0
  Constant3 : Constant  Value=uS0
  Constant4 : Constant  Value=tStepUp
  Constant5 : Constant  OutDataTypeStr=boolean
  Constant6 : Constant  Value=tStepDown
  Constant7 : Constant  Value=DB
  Display : Display
  VCC_Step_Function_Validation : Subsystem  TreatAsAtomicUnit=on
  u_Ref [-] : Outport  VectorParamsAs1DForOutWhenUnconnected=off
  tag scope: uS_sp=global
  wires:
  VCC_Step_Function_Validation/2(EndTime) -> Display/1
  Constant4/1 -> VCC_Step_Function_Validation/7(tHold1)
  Constant1/1 -> VCC_Step_Function_Validation/8(tStep)
  Clock/1 -> VCC_Step_Function_Validation/5(CyclicTime)
  Constant2/1 -> VCC_Step_Function_Validation/6(t0)
  Constant15/1 -> VCC_Step_Function_Validation/2(u_SP)
  VCC_Step_Function_Validation/1(u_ref) -> u_Ref [-]/1, [uS_sp]  "pB_tfs [Pa]"
  Constant3/1 -> VCC_Step_Function_Validation/3(uS0)
  Constant6/1 -> VCC_Step_Function_Validation/9(tHold2)
  Constant5/1 -> VCC_Step_Function_Validation/1(Enable)
  Constant7/1 -> VCC_Step_Function_Validation/4(DB)

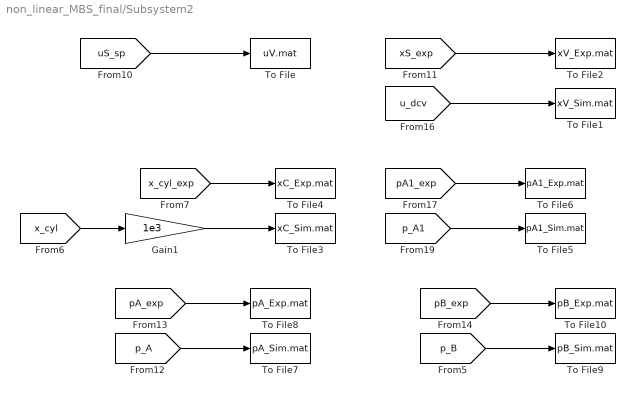
== system non_linear_MBS_final/Subsystem2 ==
  Gain1 : Gain  Gain=1e3
  To File : ToFile  Filename=uV.mat  MatrixName=uV  SaveFormat=Timeseries
  To File1 : ToFile  Filename=xV_Sim.mat  MatrixName=xV_Sim  SaveFormat=Timeseries
  To File10 : ToFile  Filename=pB_Exp.mat  MatrixName=pB_Exp  SaveFormat=Timeseries
  To File2 : ToFile  Filename=xV_Exp.mat  MatrixName=xV_Exp  SaveFormat=Timeseries
  To File3 : ToFile  Filename=xC_Sim.mat  MatrixName=xC_Sim  SaveFormat=Timeseries
  To File4 : ToFile  Filename=xC_Exp.mat  MatrixName=xC_Exp  SaveFormat=Timeseries
  To File5 : ToFile  Filename=pA1_Sim.mat  MatrixName=pA1_Sim  SaveFormat=Timeseries
  To File6 : ToFile  Filename=pA1_Exp.mat  MatrixName=pA1_Exp  SaveFormat=Timeseries
  To File7 : ToFile  Filename=pA_Sim.mat  MatrixName=pA_Sim  SaveFormat=Timeseries
  To File8 : ToFile  Filename=pA_Exp.mat  MatrixName=pA_Exp  SaveFormat=Timeseries
  To File9 : ToFile  Filename=pB_Sim.mat  MatrixName=pB_Sim  SaveFormat=Timeseries
  wires:
  [x_cyl] -> Gain1/1
  [uS_sp] -> To File/1
  [xS_exp] -> To File2/1
  [u_dcv] -> To File1/1
  Gain1/1 -> To File3/1
  [x_cyl_exp] -> To File4/1
  [pA1_exp] -> To File6/1
  [p_A1] -> To File5/1
  [pA_exp] -> To File8/1
  [p_A] -> To File7/1
  [p_B] -> To File9/1
  [pB_exp] -> To File10/1
